simulink model "chapter2_5"  (R2022a) (continued)
== system chapter2_5/Subsystem3/Subsystem/Subsystem ==
  In1 : Inport
  In2 : Inport  Port=2
  Add : Sum  Inputs=++++++++++++++++++++
  Add Constant{,1..18} (19 alike) : Bias  Bias=1  SaturateOnIntegerOverflow=off
  Subsystem1 : Subsystem
  Subsystem10 : Subsystem
  Subsystem11 : Subsystem
  Subsystem12 : Subsystem
  Subsystem13 : Subsystem
  Subsystem14 : Subsystem
  Subsystem15 : Subsystem
  Subsystem16 : Subsystem
  Subsystem17 : Subsystem
  Subsystem18 : Subsystem
  Subsystem19 : Subsystem
  Subsystem2 : Subsystem
  Subsystem20 : Subsystem
  Subsystem3 : Subsystem
  Subsystem4 : Subsystem
  Subsystem5 : Subsystem
  Subsystem6 : Subsystem
  Subsystem7 : Subsystem
  Subsystem8 : Subsystem
  Subsystem9 : Subsystem
  Out1 : Outport
  wires:
  Subsystem14/1(Out1) -> Add/9
  Add Constant9/1 -> Subsystem11/1(index), Add Constant10/1
  Add Constant2/1 -> Subsystem2/1(index), Add Constant3/1
  [Ff] -> Subsystem13/2(F)
  Add Constant11/1 -> Subsystem16/1(index), Add Constant12/1
  Add Constant14/1 -> Subsystem13/1(index), Add Constant15/1
  Subsystem8/1(Out1) -> Add/5
  [Ff] -> Subsystem17/2(F)
  Add Constant15/1 -> Subsystem20/1(index), Add Constant16/1
  Subsystem1/1(Out1) -> Add/2
  Add Constant/1 -> Subsystem1/1(index), Add Constant1/1
  Add Constant3/1 -> Subsystem8/1(index), Add Constant4/1
  Subsystem9/1(Out1) -> Add/10
  [Ff] -> Subsystem3/2(F)
  Add Constant1/1 -> Subsystem3/1(index), Add Constant2/1
  Subsystem7/1(Out1) -> Add/7
  Add Constant17/1 -> Subsystem19/1(index), Add Constant18/1
  Add Constant18/1 -> Subsystem18/1(index)
  [Ff] -> Subsystem15/2(F)
  [Ff] -> Subsystem10/2(F)
  Subsystem5/1(Out1) -> Add/8
  Subsystem10/1(Out1) -> Add/12
  Subsystem6/1(Out1) -> Add/1
  Add Constant16/1 -> Subsystem17/1(index), Add Constant17/1
  Add Constant10/1 -> Subsystem10/1(index), Add Constant11/1
  [Ff] -> Subsystem20/2(F)
  Subsystem17/1(Out1) -> Add/18
  Subsystem19/1(Out1) -> Add/19
  [Ff] -> Subsystem1/2(F)
  [Ff] -> Subsystem19/2(F)
  Add Constant8/1 -> Subsystem9/1(index), Add Constant9/1
  [Ff] -> Subsystem2/2(F)
  Subsystem3/1(Out1) -> Add/3
  [Ff] -> Subsystem8/2(F)
  Subsystem20/1(Out1) -> Add/17
  Subsystem12/1(Out1) -> Add/14
  In1/1 -> Subsystem6/1(index), Add Constant/1
  Subsystem16/1(Out1) -> Add/13
  Subsystem13/1(Out1) -> Add/16
  [Ff] -> Subsystem6/2(F)
  [Ff] -> Subsystem7/2(F)
  [Ff] -> Subsystem11/2(F)
  Subsystem15/1(Out1) -> Add/15
  Add Constant13/1 -> Subsystem15/1(index), Add Constant14/1
  Subsystem2/1(Out1) -> Add/4
  Add Constant12/1 -> Subsystem12/1(index), Add Constant13/1
  Subsystem4/1(Out1) -> Add/6
  [Ff] -> Subsystem4/2(F)
  [Ff] -> Subsystem9/2(F)
  [Ff] -> Subsystem5/2(F)
  [Ff] -> Subsystem14/2(F)
  [Ff] -> Subsystem12/2(F)
  Subsystem18/1(Out1) -> Add/20
  Subsystem11/1(Out1) -> Add/11
  Add Constant5/1 -> Subsystem7/1(index), Add Constant6/1
  Add Constant6/1 -> Subsystem5/1(index), Add Constant7/1
  Add Constant4/1 -> Subsystem4/1(index), Add Constant5/1
  Add Constant7/1 -> Subsystem14/1(index), Add Constant8/1
  [Ff] -> Subsystem16/2(F)
  [Ff] -> Subsystem18/2(F)
  Add/1 -> Out1/1
  In2/1 -> [Ff]

== system chapter2_5/Subsystem3/Subsystem/Subsystem1 ==
  In1 : Inport
  In2 : Inport  Port=2
  Add : Sum  Inputs=++++++++++++++++++++
  Add Constant{,1..18} (19 alike) : Bias  Bias=1  SaturateOnIntegerOverflow=off
  Subsystem1 : Subsystem
  Subsystem10 : Subsystem
  Subsystem11 : Subsystem
  Subsystem12 : Subsystem
  Subsystem13 : Subsystem
  Subsystem14 : Subsystem
  Subsystem15 : Subsystem
  Subsystem16 : Subsystem
  Subsystem17 : Subsystem
  Subsystem18 : Subsystem
  Subsystem19 : Subsystem
  Subsystem2 : Subsystem
  Subsystem20 : Subsystem
  Subsystem3 : Subsystem
  Subsystem4 : Subsystem
  Subsystem5 : Subsystem
  Subsystem6 : Subsystem
  Subsystem7 : Subsystem
  Subsystem8 : Subsystem
  Subsystem9 : Subsystem
  Out1 : Outport
  wires:
  In2/1 -> [Ff]
  Add/1 -> Out1/1
  [Ff] -> Subsystem18/2(F)
  [Ff] -> Subsystem16/2(F)
  Add Constant7/1 -> Add Constant8/1, Subsystem14/1(index)
  Add Constant4/1 -> Add Constant5/1, Subsystem4/1(index)
  Add Constant6/1 -> Add Constant7/1, Subsystem5/1(index)
  Add Constant5/1 -> Add Constant6/1, Subsystem7/1(index)
  Subsystem11/1(Out1) -> Add/11
  Subsystem18/1(Out1) -> Add/20
  [Ff] -> Subsystem12/2(F)
  [Ff] -> Subsystem14/2(F)
  [Ff] -> Subsystem5/2(F)
  [Ff] -> Subsystem9/2(F)
  [Ff] -> Subsystem4/2(F)
  Subsystem4/1(Out1) -> Add/6
  Add Constant12/1 -> Add Constant13/1, Subsystem12/1(index)
  Subsystem2/1(Out1) -> Add/4
  Add Constant13/1 -> Add Constant14/1, Subsystem15/1(index)
  Subsystem15/1(Out1) -> Add/15
  [Ff] -> Subsystem11/2(F)
  [Ff] -> Subsystem7/2(F)
  [Ff] -> Subsystem6/2(F)
  Subsystem13/1(Out1) -> Add/16
  Subsystem16/1(Out1) -> Add/13
  In1/1 -> Add Constant/1, Subsystem6/1(index)
  Subsystem12/1(Out1) -> Add/14
  Subsystem20/1(Out1) -> Add/17
  [Ff] -> Subsystem8/2(F)
  Subsystem3/1(Out1) -> Add/3
  [Ff] -> Subsystem2/2(F)
  Add Constant8/1 -> Add Constant9/1, Subsystem9/1(index)
  [Ff] -> Subsystem19/2(F)
  [Ff] -> Subsystem1/2(F)
  Subsystem19/1(Out1) -> Add/19
  Subsystem17/1(Out1) -> Add/18
  [Ff] -> Subsystem20/2(F)
  Add Constant10/1 -> Add Constant11/1, Subsystem10/1(index)
  Add Constant16/1 -> Add Constant17/1, Subsystem17/1(index)
  Subsystem6/1(Out1) -> Add/1
  Subsystem10/1(Out1) -> Add/12
  Subsystem5/1(Out1) -> Add/8
  [Ff] -> Subsystem10/2(F)
  [Ff] -> Subsystem15/2(F)
  Add Constant18/1 -> Subsystem18/1(index)
  Add Constant17/1 -> Add Constant18/1, Subsystem19/1(index)
  Subsystem7/1(Out1) -> Add/7
  Add Constant1/1 -> Add Constant2/1, Subsystem3/1(index)
  [Ff] -> Subsystem3/2(F)
  Subsystem9/1(Out1) -> Add/10
  Add Constant3/1 -> Add Constant4/1, Subsystem8/1(index)
  Add Constant/1 -> Add Constant1/1, Subsystem1/1(index)
  Subsystem1/1(Out1) -> Add/2
  Add Constant15/1 -> Add Constant16/1, Subsystem20/1(index)
  [Ff] -> Subsystem17/2(F)
  Subsystem8/1(Out1) -> Add/5
  Add Constant14/1 -> Add Constant15/1, Subsystem13/1(index)
  Add Constant11/1 -> Add Constant12/1, Subsystem16/1(index)
  [Ff] -> Subsystem13/2(F)
  Add Constant2/1 -> Add Constant3/1, Subsystem2/1(index)
  Add Constant9/1 -> Add Constant10/1, Subsystem11/1(index)
  Subsystem14/1(Out1) -> Add/9

== system chapter2_5/Subsystem3/Subsystem/Subsystem2 ==
  In1 : Inport
  In2 : Inport  Port=2
  Add : Sum  Inputs=++++++++++++++++++++
  Add Constant{,1..18} (19 alike) : Bias  Bias=1  SaturateOnIntegerOverflow=off
  Subsystem1 : Subsystem
  Subsystem10 : Subsystem
  Subsystem11 : Subsystem
  Subsystem12 : Subsystem
  Subsystem13 : Subsystem
  Subsystem14 : Subsystem
  Subsystem15 : Subsystem
  Subsystem16 : Subsystem
  Subsystem17 : Subsystem
  Subsystem18 : Subsystem
  Subsystem19 : Subsystem
  Subsystem2 : Subsystem
  Subsystem20 : Subsystem
  Subsystem3 : Subsystem
  Subsystem4 : Subsystem
  Subsystem5 : Subsystem
  Subsystem6 : Subsystem
  Subsystem7 : Subsystem
  Subsystem8 : Subsystem
  Subsystem9 : Subsystem
  Out1 : Outport
  wires:
  Subsystem14/1(Out1) -> Add/9
  Add Constant9/1 -> Subsystem11/1(index), Add Constant10/1
  Add Constant2/1 -> Subsystem2/1(index), Add Constant3/1
  [Ff] -> Subsystem13/2(F)
  Add Constant11/1 -> Subsystem16/1(index), Add Constant12/1
  Add Constant14/1 -> Subsystem13/1(index), Add Constant15/1
  Subsystem8/1(Out1) -> Add/5
  [Ff] -> Subsystem17/2(F)
  Add Constant15/1 -> Subsystem20/1(index), Add Constant16/1
  Subsystem1/1(Out1) -> Add/2
  Add Constant/1 -> Subsystem1/1(index), Add Constant1/1
  Add Constant3/1 -> Subsystem8/1(index), Add Constant4/1
  Subsystem9/1(Out1) -> Add/10
  [Ff] -> Subsystem3/2(F)
  Add Constant1/1 -> Subsystem3/1(index), Add Constant2/1
  Subsystem7/1(Out1) -> Add/7
  Add Constant17/1 -> Subsystem19/1(index), Add Constant18/1
  Add Constant18/1 -> Subsystem18/1(index)
  [Ff] -> Subsystem15/2(F)
  [Ff] -> Subsystem10/2(F)
  Subsystem5/1(Out1) -> Add/8
  Subsystem10/1(Out1) -> Add/12
  Subsystem6/1(Out1) -> Add/1
  Add Constant16/1 -> Subsystem17/1(index), Add Constant17/1
  Add Constant10/1 -> Subsystem10/1(index), Add Constant11/1
  [Ff] -> Subsystem20/2(F)
  Subsystem17/1(Out1) -> Add/18
  Subsystem19/1(Out1) -> Add/19
  [Ff] -> Subsystem1/2(F)
  [Ff] -> Subsystem19/2(F)
  Add Constant8/1 -> Subsystem9/1(index), Add Constant9/1
  [Ff] -> Subsystem2/2(F)
  Subsystem3/1(Out1) -> Add/3
  [Ff] -> Subsystem8/2(F)
  Subsystem20/1(Out1) -> Add/17
  Subsystem12/1(Out1) -> Add/14
  In1/1 -> Subsystem6/1(index), Add Constant/1
  Subsystem16/1(Out1) -> Add/13
  Subsystem13/1(Out1) -> Add/16
  [Ff] -> Subsystem6/2(F)
  [Ff] -> Subsystem7/2(F)
  [Ff] -> Subsystem11/2(F)
  Subsystem15/1(Out1) -> Add/15
  Add Constant13/1 -> Subsystem15/1(index), Add Constant14/1
  Subsystem2/1(Out1) -> Add/4
  Add Constant12/1 -> Subsystem12/1(index), Add Constant13/1
  Subsystem4/1(Out1) -> Add/6
  [Ff] -> Subsystem4/2(F)
  [Ff] -> Subsystem9/2(F)
  [Ff] -> Subsystem5/2(F)
  [Ff] -> Subsystem14/2(F)
  [Ff] -> Subsystem12/2(F)
  Subsystem18/1(Out1) -> Add/20
  Subsystem11/1(Out1) -> Add/11
  Add Constant5/1 -> Subsystem7/1(index), Add Constant6/1
  Add Constant6/1 -> Subsystem5/1(index), Add Constant7/1
  Add Constant4/1 -> Subsystem4/1(index), Add Constant5/1
  Add Constant7/1 -> Subsystem14/1(index), Add Constant8/1
  [Ff] -> Subsystem16/2(F)
  [Ff] -> Subsystem18/2(F)
  Add/1 -> Out1/1
  In2/1 -> [Ff]

== system chapter2_5/Subsystem3/Subsystem/Subsystem3 ==
  In1 : Inport
  In2 : Inport  Port=2
  Add : Sum  Inputs=++++++++++++++++++++
  Add Constant{,1..18} (19 alike) : Bias  Bias=1  SaturateOnIntegerOverflow=off
  Subsystem1 : Subsystem
  Subsystem10 : Subsystem
  Subsystem11 : Subsystem
  Subsystem12 : Subsystem
  Subsystem13 : Subsystem
  Subsystem14 : Subsystem
  Subsystem15 : Subsystem
  Subsystem16 : Subsystem
  Subsystem17 : Subsystem
  Subsystem18 : Subsystem
  Subsystem19 : Subsystem
  Subsystem2 : Subsystem
  Subsystem20 : Subsystem
  Subsystem3 : Subsystem
  Subsystem4 : Subsystem
  Subsystem5 : Subsystem
  Subsystem6 : Subsystem
  Subsystem7 : Subsystem
  Subsystem8 : Subsystem
  Subsystem9 : Subsystem
  Out1 : Outport
  wires:
  In2/1 -> [Ff]
  Add/1 -> Out1/1
  [Ff] -> Subsystem18/2(F)
  [Ff] -> Subsystem16/2(F)
  Add Constant7/1 -> Add Constant8/1, Subsystem14/1(index)
  Add Constant4/1 -> Add Constant5/1, Subsystem4/1(index)
  Add Constant6/1 -> Add Constant7/1, Subsystem5/1(index)
  Add Constant5/1 -> Add Constant6/1, Subsystem7/1(index)
  Subsystem11/1(Out1) -> Add/11
  Subsystem18/1(Out1) -> Add/20
  [Ff] -> Subsystem12/2(F)
  [Ff] -> Subsystem14/2(F)
  [Ff] -> Subsystem5/2(F)
  [Ff] -> Subsystem9/2(F)
  [Ff] -> Subsystem4/2(F)
  Subsystem4/1(Out1) -> Add/6
  Add Constant12/1 -> Add Constant13/1, Subsystem12/1(index)
  Subsystem2/1(Out1) -> Add/4
  Add Constant13/1 -> Add Constant14/1, Subsystem15/1(index)
  Subsystem15/1(Out1) -> Add/15
  [Ff] -> Subsystem11/2(F)
  [Ff] -> Subsystem7/2(F)
  [Ff] -> Subsystem6/2(F)
  Subsystem13/1(Out1) -> Add/16
  Subsystem16/1(Out1) -> Add/13
  In1/1 -> Add Constant/1, Subsystem6/1(index)
  Subsystem12/1(Out1) -> Add/14
  Subsystem20/1(Out1) -> Add/17
  [Ff] -> Subsystem8/2(F)
  Subsystem3/1(Out1) -> Add/3
  [Ff] -> Subsystem2/2(F)
  Add Constant8/1 -> Add Constant9/1, Subsystem9/1(index)
  [Ff] -> Subsystem19/2(F)
  [Ff] -> Subsystem1/2(F)
  Subsystem19/1(Out1) -> Add/19
  Subsystem17/1(Out1) -> Add/18
  [Ff] -> Subsystem20/2(F)
  Add Constant10/1 -> Add Constant11/1, Subsystem10/1(index)
  Add Constant16/1 -> Add Constant17/1, Subsystem17/1(index)
  Subsystem6/1(Out1) -> Add/1
  Subsystem10/1(Out1) -> Add/12
  Subsystem5/1(Out1) -> Add/8
  [Ff] -> Subsystem10/2(F)
  [Ff] -> Subsystem15/2(F)
  Add Constant18/1 -> Subsystem18/1(index)
  Add Constant17/1 -> Add Constant18/1, Subsystem19/1(index)
  Subsystem7/1(Out1) -> Add/7
  Add Constant1/1 -> Add Constant2/1, Subsystem3/1(index)
  [Ff] -> Subsystem3/2(F)
  Subsystem9/1(Out1) -> Add/10
  Add Constant3/1 -> Add Constant4/1, Subsystem8/1(index)
  Add Constant/1 -> Add Constant1/1, Subsystem1/1(index)
  Subsystem1/1(Out1) -> Add/2
  Add Constant15/1 -> Add Constant16/1, Subsystem20/1(index)
  [Ff] -> Subsystem17/2(F)
  Subsystem8/1(Out1) -> Add/5
  Add Constant14/1 -> Add Constant15/1, Subsystem13/1(index)
  Add Constant11/1 -> Add Constant12/1, Subsystem16/1(index)
  [Ff] -> Subsystem13/2(F)
  Add Constant2/1 -> Add Constant3/1, Subsystem2/1(index)
  Add Constant9/1 -> Add Constant10/1, Subsystem11/1(index)
  Subsystem14/1(Out1) -> Add/9

== system chapter2_5/Subsystem3/Subsystem/Subsystem4 ==
  In1 : Inport
  In2 : Inport  Port=2
  Add : Sum  Inputs=++++++++++++++++++++
  Add Constant{,1..18} (19 alike) : Bias  Bias=1  SaturateOnIntegerOverflow=off
  Subsystem1 : Subsystem
  Subsystem10 : Subsystem
  Subsystem11 : Subsystem
  Subsystem12 : Subsystem
  Subsystem13 : Subsystem
  Subsystem14 : Subsystem
  Subsystem15 : Subsystem
  Subsystem16 : Subsystem
  Subsystem17 : Subsystem
  Subsystem18 : Subsystem
  Subsystem19 : Subsystem
  Subsystem2 : Subsystem
  Subsystem20 : Subsystem
  Subsystem3 : Subsystem
  Subsystem4 : Subsystem
  Subsystem5 : Subsystem
  Subsystem6 : Subsystem
  Subsystem7 : Subsystem
  Subsystem8 : Subsystem
  Subsystem9 : Subsystem
  Out1 : Outport
  wires:
  Subsystem14/1(Out1) -> Add/9
  Add Constant9/1 -> Subsystem11/1(index), Add Constant10/1
  Add Constant2/1 -> Subsystem2/1(index), Add Constant3/1
  [Ff] -> Subsystem13/2(F)
  Add Constant11/1 -> Subsystem16/1(index), Add Constant12/1
  Add Constant14/1 -> Subsystem13/1(index), Add Constant15/1
  Subsystem8/1(Out1) -> Add/5
  [Ff] -> Subsystem17/2(F)
  Add Constant15/1 -> Subsystem20/1(index), Add Constant16/1
  Subsystem1/1(Out1) -> Add/2
  Add Constant/1 -> Subsystem1/1(index), Add Constant1/1
  Add Constant3/1 -> Subsystem8/1(index), Add Constant4/1
  Subsystem9/1(Out1) -> Add/10
  [Ff] -> Subsystem3/2(F)
  Add Constant1/1 -> Subsystem3/1(index), Add Constant2/1
  Subsystem7/1(Out1) -> Add/7
  Add Constant17/1 -> Subsystem19/1(index), Add Constant18/1
  Add Constant18/1 -> Subsystem18/1(index)
  [Ff] -> Subsystem15/2(F)
  [Ff] -> Subsystem10/2(F)
  Subsystem5/1(Out1) -> Add/8
  Subsystem10/1(Out1) -> Add/12
  Subsystem6/1(Out1) -> Add/1
  Add Constant16/1 -> Subsystem17/1(index), Add Constant17/1
  Add Constant10/1 -> Subsystem10/1(index), Add Constant11/1
  [Ff] -> Subsystem20/2(F)
  Subsystem17/1(Out1) -> Add/18
  Subsystem19/1(Out1) -> Add/19
  [Ff] -> Subsystem1/2(F)
  [Ff] -> Subsystem19/2(F)
  Add Constant8/1 -> Subsystem9/1(index), Add Constant9/1
  [Ff] -> Subsystem2/2(F)
  Subsystem3/1(Out1) -> Add/3
  [Ff] -> Subsystem8/2(F)
  Subsystem20/1(Out1) -> Add/17
  Subsystem12/1(Out1) -> Add/14
  In1/1 -> Subsystem6/1(index), Add Constant/1
  Subsystem16/1(Out1) -> Add/13
  Subsystem13/1(Out1) -> Add/16
  [Ff] -> Subsystem6/2(F)
  [Ff] -> Subsystem7/2(F)
  [Ff] -> Subsystem11/2(F)
  Subsystem15/1(Out1) -> Add/15
  Add Constant13/1 -> Subsystem15/1(index), Add Constant14/1
  Subsystem2/1(Out1) -> Add/4
  Add Constant12/1 -> Subsystem12/1(index), Add Constant13/1
  Subsystem4/1(Out1) -> Add/6
  [Ff] -> Subsystem4/2(F)
  [Ff] -> Subsystem9/2(F)
  [Ff] -> Subsystem5/2(F)
  [Ff] -> Subsystem14/2(F)
  [Ff] -> Subsystem12/2(F)
  Subsystem18/1(Out1) -> Add/20
  Subsystem11/1(Out1) -> Add/11
  Add Constant5/1 -> Subsystem7/1(index), Add Constant6/1
  Add Constant6/1 -> Subsystem5/1(index), Add Constant7/1
  Add Constant4/1 -> Subsystem4/1(index), Add Constant5/1
  Add Constant7/1 -> Subsystem14/1(index), Add Constant8/1
  [Ff] -> Subsystem16/2(F)
  [Ff] -> Subsystem18/2(F)
  Add/1 -> Out1/1
  In2/1 -> [Ff]

== system chapter2_5/Subsystem3/Subsystem/Subsystem5 ==
  In1 : Inport
  In2 : Inport  Port=2
  Add : Sum  Inputs=++++++++++++++++++++
  Add Constant{,1..18} (19 alike) : Bias  Bias=1  SaturateOnIntegerOverflow=off
  Subsystem1 : Subsystem
  Subsystem10 : Subsystem
  Subsystem11 : Subsystem
  Subsystem12 : Subsystem
  Subsystem13 : Subsystem
  Subsystem14 : Subsystem
  Subsystem15 : Subsystem
  Subsystem16 : Subsystem
  Subsystem17 : Subsystem
  Subsystem18 : Subsystem
  Subsystem19 : Subsystem
  Subsystem2 : Subsystem
  Subsystem20 : Subsystem
  Subsystem3 : Subsystem
  Subsystem4 : Subsystem
  Subsystem5 : Subsystem
  Subsystem6 : Subsystem
  Subsystem7 : Subsystem
  Subsystem8 : Subsystem
  Subsystem9 : Subsystem
  Out1 : Outport
  wires:
  In2/1 -> [Ff]
  Add/1 -> Out1/1
  [Ff] -> Subsystem18/2(F)
  [Ff] -> Subsystem16/2(F)
  Add Constant7/1 -> Add Constant8/1, Subsystem14/1(index)
  Add Constant4/1 -> Add Constant5/1, Subsystem4/1(index)
  Add Constant6/1 -> Add Constant7/1, Subsystem5/1(index)
  Add Constant5/1 -> Add Constant6/1, Subsystem7/1(index)
  Subsystem11/1(Out1) -> Add/11
  Subsystem18/1(Out1) -> Add/20
  [Ff] -> Subsystem12/2(F)
  [Ff] -> Subsystem14/2(F)
  [Ff] -> Subsystem5/2(F)
  [Ff] -> Subsystem9/2(F)
  [Ff] -> Subsystem4/2(F)
  Subsystem4/1(Out1) -> Add/6
  Add Constant12/1 -> Add Constant13/1, Subsystem12/1(index)
  Subsystem2/1(Out1) -> Add/4
  Add Constant13/1 -> Add Constant14/1, Subsystem15/1(index)
  Subsystem15/1(Out1) -> Add/15
  [Ff] -> Subsystem11/2(F)
  [Ff] -> Subsystem7/2(F)
  [Ff] -> Subsystem6/2(F)
  Subsystem13/1(Out1) -> Add/16
  Subsystem16/1(Out1) -> Add/13
  In1/1 -> Add Constant/1, Subsystem6/1(index)
  Subsystem12/1(Out1) -> Add/14
  Subsystem20/1(Out1) -> Add/17
  [Ff] -> Subsystem8/2(F)
  Subsystem3/1(Out1) -> Add/3
  [Ff] -> Subsystem2/2(F)
  Add Constant8/1 -> Add Constant9/1, Subsystem9/1(index)
  [Ff] -> Subsystem19/2(F)
  [Ff] -> Subsystem1/2(F)
  Subsystem19/1(Out1) -> Add/19
  Subsystem17/1(Out1) -> Add/18
  [Ff] -> Subsystem20/2(F)
  Add Constant10/1 -> Add Constant11/1, Subsystem10/1(index)
  Add Constant16/1 -> Add Constant17/1, Subsystem17/1(index)
  Subsystem6/1(Out1) -> Add/1
  Subsystem10/1(Out1) -> Add/12
  Subsystem5/1(Out1) -> Add/8
  [Ff] -> Subsystem10/2(F)
  [Ff] -> Subsystem15/2(F)
  Add Constant18/1 -> Subsystem18/1(index)
  Add Constant17/1 -> Add Constant18/1, Subsystem19/1(index)
  Subsystem7/1(Out1) -> Add/7
  Add Constant1/1 -> Add Constant2/1, Subsystem3/1(index)
  [Ff] -> Subsystem3/2(F)
  Subsystem9/1(Out1) -> Add/10
  Add Constant3/1 -> Add Constant4/1, Subsystem8/1(index)
  Add Constant/1 -> Add Constant1/1, Subsystem1/1(index)
  Subsystem1/1(Out1) -> Add/2
  Add Constant15/1 -> Add Constant16/1, Subsystem20/1(index)
  [Ff] -> Subsystem17/2(F)
  Subsystem8/1(Out1) -> Add/5
  Add Constant14/1 -> Add Constant15/1, Subsystem13/1(index)
  Add Constant11/1 -> Add Constant12/1, Subsystem16/1(index)
  [Ff] -> Subsystem13/2(F)
  Add Constant2/1 -> Add Constant3/1, Subsystem2/1(index)
  Add Constant9/1 -> Add Constant10/1, Subsystem11/1(index)
  Subsystem14/1(Out1) -> Add/9

== system chapter2_5/Subsystem3/Subsystem/Subsystem6 ==
  In1 : Inport
  In2 : Inport  Port=2
  Add : Sum  Inputs=++++++++++++++++++++
  Add Constant{,1..18} (19 alike) : Bias  Bias=1  SaturateOnIntegerOverflow=off
  Subsystem1 : Subsystem
  Subsystem10 : Subsystem
  Subsystem11 : Subsystem
  Subsystem12 : Subsystem
  Subsystem13 : Subsystem
  Subsystem14 : Subsystem
  Subsystem15 : Subsystem
  Subsystem16 : Subsystem
  Subsystem17 : Subsystem
  Subsystem18 : Subsystem
  Subsystem19 : Subsystem
  Subsystem2 : Subsystem
  Subsystem20 : Subsystem
  Subsystem3 : Subsystem
  Subsystem4 : Subsystem
  Subsystem5 : Subsystem
  Subsystem6 : Subsystem
  Subsystem7 : Subsystem
  Subsystem8 : Subsystem
  Subsystem9 : Subsystem
  Out1 : Outport
  wires:
  Subsystem14/1(Out1) -> Add/9
  Add Constant9/1 -> Subsystem11/1(index), Add Constant10/1
  Add Constant2/1 -> Subsystem2/1(index), Add Constant3/1
  [Ff] -> Subsystem13/2(F)
  Add Constant11/1 -> Subsystem16/1(index), Add Constant12/1
  Add Constant14/1 -> Subsystem13/1(index), Add Constant15/1
  Subsystem8/1(Out1) -> Add/5
  [Ff] -> Subsystem17/2(F)
  Add Constant15/1 -> Subsystem20/1(index), Add Constant16/1
  Subsystem1/1(Out1) -> Add/2
  Add Constant/1 -> Subsystem1/1(index), Add Constant1/1
  Add Constant3/1 -> Subsystem8/1(index), Add Constant4/1
  Subsystem9/1(Out1) -> Add/10
  [Ff] -> Subsystem3/2(F)
  Add Constant1/1 -> Subsystem3/1(index), Add Constant2/1
  Subsystem7/1(Out1) -> Add/7
  Add Constant17/1 -> Subsystem19/1(index), Add Constant18/1
  Add Constant18/1 -> Subsystem18/1(index)
  [Ff] -> Subsystem15/2(F)
  [Ff] -> Subsystem10/2(F)
  Subsystem5/1(Out1) -> Add/8
  Subsystem10/1(Out1) -> Add/12
  Subsystem6/1(Out1) -> Add/1
  Add Constant16/1 -> Subsystem17/1(index), Add Constant17/1
  Add Constant10/1 -> Subsystem10/1(index), Add Constant11/1
  [Ff] -> Subsystem20/2(F)
  Subsystem17/1(Out1) -> Add/18
  Subsystem19/1(Out1) -> Add/19
  [Ff] -> Subsystem1/2(F)
  [Ff] -> Subsystem19/2(F)
  Add Constant8/1 -> Subsystem9/1(index), Add Constant9/1
  [Ff] -> Subsystem2/2(F)
  Subsystem3/1(Out1) -> Add/3
  [Ff] -> Subsystem8/2(F)
  Subsystem20/1(Out1) -> Add/17
  Subsystem12/1(Out1) -> Add/14
  In1/1 -> Subsystem6/1(index), Add Constant/1
  Subsystem16/1(Out1) -> Add/13
  Subsystem13/1(Out1) -> Add/16
  [Ff] -> Subsystem6/2(F)
  [Ff] -> Subsystem7/2(F)
  [Ff] -> Subsystem11/2(F)
  Subsystem15/1(Out1) -> Add/15
  Add Constant13/1 -> Subsystem15/1(index), Add Constant14/1
  Subsystem2/1(Out1) -> Add/4
  Add Constant12/1 -> Subsystem12/1(index), Add Constant13/1
  Subsystem4/1(Out1) -> Add/6
  [Ff] -> Subsystem4/2(F)
  [Ff] -> Subsystem9/2(F)
  [Ff] -> Subsystem5/2(F)
  [Ff] -> Subsystem14/2(F)
  [Ff] -> Subsystem12/2(F)
  Subsystem18/1(Out1) -> Add/20
  Subsystem11/1(Out1) -> Add/11
  Add Constant5/1 -> Subsystem7/1(index), Add Constant6/1
  Add Constant6/1 -> Subsystem5/1(index), Add Constant7/1
  Add Constant4/1 -> Subsystem4/1(index), Add Constant5/1
  Add Constant7/1 -> Subsystem14/1(index), Add Constant8/1
  [Ff] -> Subsystem16/2(F)
  [Ff] -> Subsystem18/2(F)
  Add/1 -> Out1/1
  In2/1 -> [Ff]

== system chapter2_5/Subsystem3/Subsystem1/Subsystem ==
  In1 : Inport
  In2 : Inport  Port=2
  Add : Sum  Inputs=++++++++++++++++++++
  Add Constant{,1..18} (19 alike) : Bias  Bias=1  SaturateOnIntegerOverflow=off
  Subsystem1 : Subsystem
  Subsystem10 : Subsystem
  Subsystem11 : Subsystem
  Subsystem12 : Subsystem
  Subsystem13 : Subsystem
  Subsystem14 : Subsystem
  Subsystem15 : Subsystem
  Subsystem16 : Subsystem
  Subsystem17 : Subsystem
  Subsystem18 : Subsystem
  Subsystem19 : Subsystem
  Subsystem2 : Subsystem
  Subsystem20 : Subsystem
  Subsystem3 : Subsystem
  Subsystem4 : Subsystem
  Subsystem5 : Subsystem
  Subsystem6 : Subsystem
  Subsystem7 : Subsystem
  Subsystem8 : Subsystem
  Subsystem9 : Subsystem
  Out1 : Outport
  wires:
  Add Constant7/1 -> Subsystem13/1(index), Add Constant8/1
  Subsystem15/1(Out1) -> Add/15
  [Ff] -> Subsystem3/2(F)
  Add Constant14/1 -> Subsystem12/1(index), Add Constant15/1
  Subsystem8/1(Out1) -> Add/5
  [Ff] -> Subsystem7/2(F)
  Add Constant8/1 -> Subsystem9/1(index), Add Constant9/1
  Subsystem9/1(Out1) -> Add/10
  [Ff] -> Subsystem6/2(F)
  Add Constant4/1 -> Subsystem4/1(index), Add Constant5/1
  Subsystem18/1(Out1) -> Add/20
  Add Constant15/1 -> Subsystem15/1(index), Add Constant16/1
  Subsystem4/1(Out1) -> Add/6
  Add Constant6/1 -> Subsystem6/1(index), Add Constant7/1
  Subsystem16/1(Out1) -> Add/13
  In1/1 -> Add Constant/1, Subsystem5/1(index)
  Subsystem20/1(Out1) -> Add/17
  [Ff] -> Subsystem17/2(F)
  [Ff] -> Subsystem10/2(F)
  Add Constant10/1 -> Subsystem10/1(index), Add Constant11/1
  Add Constant12/1 -> Subsystem17/1(index), Add Constant13/1
  Add Constant3/1 -> Subsystem8/1(index), Add Constant4/1
  Add Constant/1 -> Subsystem1/1(index), Add Constant1/1
  [Ff] -> Subsystem11/2(F)
  Subsystem11/1(Out1) -> Add/11
  [Ff] -> Subsystem19/2(F)
  Subsystem5/1(Out1) -> Add/1
  [Ff] -> Subsystem15/2(F)
  Subsystem1/1(Out1) -> Add/2
  Add Constant13/1 -> Subsystem19/1(index), Add Constant18/1
  [Ff] -> Subsystem9/2(F)
  Add Constant1/1 -> Subsystem3/1(index), Add Constant2/1
  Subsystem10/1(Out1) -> Add/12
  Add Constant2/1 -> Subsystem2/1(index), Add Constant3/1
  [Ff] -> Subsystem2/2(F)
  Subsystem12/1(Out1) -> Add/14
  [Ff] -> Subsystem18/2(F)
  [Ff] -> Subsystem4/2(F)
  Subsystem17/1(Out1) -> Add/18
  Subsystem13/1(Out1) -> Add/9
  [Ff] -> Subsystem1/2(F)
  [Ff] -> Subsystem16/2(F)
  Subsystem14/1(Out1) -> Add/16
  [Ff] -> Subsystem5/2(F)
  [Ff] -> Subsystem20/2(F)
  Subsystem3/1(Out1) -> Add/3
  Subsystem7/1(Out1) -> Add/7
  Add/1 -> Out1/1
  [Ff] -> Subsystem14/2(F)
  [Ff] -> Subsystem8/2(F)
  Subsystem19/1(Out1) -> Add/19
  [Ff] -> Subsystem12/2(F)
  [Ff] -> Subsystem13/2(F)
  Add Constant9/1 -> Subsystem11/1(index), Add Constant10/1
  Add Constant5/1 -> Subsystem7/1(index), Add Constant6/1
  Subsystem6/1(Out1) -> Add/8
  Add Constant11/1 -> Subsystem16/1(index), Add Constant14/1
  Subsystem2/1(Out1) -> Add/4
  Add Constant16/1 -> Subsystem14/1(index), Add Constant17/1
  Add Constant17/1 -> Subsystem20/1(index), Add Constant12/1
  Add Constant18/1 -> Subsystem18/1(index)
  In2/1 -> [Ff]

== system chapter2_5/Subsystem3/Subsystem1/Subsystem1 ==
  In1 : Inport
  In2 : Inport  Port=2
  Add : Sum  Inputs=++++++++++++++++++++
  Add Constant{,1..18} (19 alike) : Bias  Bias=1  SaturateOnIntegerOverflow=off
  Subsystem1 : Subsystem
  Subsystem10 : Subsystem
  Subsystem11 : Subsystem
  Subsystem12 : Subsystem
  Subsystem13 : Subsystem
  Subsystem14 : Subsystem
  Subsystem15 : Subsystem
  Subsystem16 : Subsystem
  Subsystem17 : Subsystem
  Subsystem18 : Subsystem
  Subsystem19 : Subsystem
  Subsystem2 : Subsystem
  Subsystem20 : Subsystem
  Subsystem3 : Subsystem
  Subsystem4 : Subsystem
  Subsystem5 : Subsystem
  Subsystem6 : Subsystem
  Subsystem7 : Subsystem
  Subsystem8 : Subsystem
  Subsystem9 : Subsystem
  Out1 : Outport
  wires:
  In2/1 -> [Ff]
  Add Constant18/1 -> Subsystem18/1(index)
  Add Constant17/1 -> Add Constant12/1, Subsystem20/1(index)
  Add Constant16/1 -> Add Constant17/1, Subsystem14/1(index)
  Subsystem2/1(Out1) -> Add/4
  Add Constant11/1 -> Add Constant14/1, Subsystem16/1(index)
  Subsystem6/1(Out1) -> Add/8
  Add Constant5/1 -> Add Constant6/1, Subsystem7/1(index)
  Add Constant9/1 -> Add Constant10/1, Subsystem11/1(index)
  [Ff] -> Subsystem13/2(F)
  [Ff] -> Subsystem12/2(F)
  Subsystem19/1(Out1) -> Add/19
  [Ff] -> Subsystem8/2(F)
  [Ff] -> Subsystem14/2(F)
  Add/1 -> Out1/1
  Subsystem7/1(Out1) -> Add/7
  Subsystem3/1(Out1) -> Add/3
  [Ff] -> Subsystem20/2(F)
  [Ff] -> Subsystem5/2(F)
  Subsystem14/1(Out1) -> Add/16
  [Ff] -> Subsystem16/2(F)
  [Ff] -> Subsystem1/2(F)
  Subsystem13/1(Out1) -> Add/9
  Subsystem17/1(Out1) -> Add/18
  [Ff] -> Subsystem4/2(F)
  [Ff] -> Subsystem18/2(F)
  Subsystem12/1(Out1) -> Add/14
  [Ff] -> Subsystem2/2(F)
  Add Constant2/1 -> Add Constant3/1, Subsystem2/1(index)
  Subsystem10/1(Out1) -> Add/12
  Add Constant1/1 -> Add Constant2/1, Subsystem3/1(index)
  [Ff] -> Subsystem9/2(F)
  Add Constant13/1 -> Add Constant18/1, Subsystem19/1(index)
  Subsystem1/1(Out1) -> Add/2
  [Ff] -> Subsystem15/2(F)
  Subsystem5/1(Out1) -> Add/1
  [Ff] -> Subsystem19/2(F)
  Subsystem11/1(Out1) -> Add/11
  [Ff] -> Subsystem11/2(F)
  Add Constant/1 -> Add Constant1/1, Subsystem1/1(index)
  Add Constant3/1 -> Add Constant4/1, Subsystem8/1(index)
  Add Constant12/1 -> Add Constant13/1, Subsystem17/1(index)
  Add Constant10/1 -> Add Constant11/1, Subsystem10/1(index)
  [Ff] -> Subsystem10/2(F)
  [Ff] -> Subsystem17/2(F)
  Subsystem20/1(Out1) -> Add/17
  In1/1 -> Subsystem5/1(index), Add Constant/1
  Subsystem16/1(Out1) -> Add/13
  Add Constant6/1 -> Add Constant7/1, Subsystem6/1(index)
  Subsystem4/1(Out1) -> Add/6
  Add Constant15/1 -> Add Constant16/1, Subsystem15/1(index)
  Subsystem18/1(Out1) -> Add/20
  Add Constant4/1 -> Add Constant5/1, Subsystem4/1(index)
  [Ff] -> Subsystem6/2(F)
  Subsystem9/1(Out1) -> Add/10
  Add Constant8/1 -> Add Constant9/1, Subsystem9/1(index)
  [Ff] -> Subsystem7/2(F)
  Subsystem8/1(Out1) -> Add/5
  Add Constant14/1 -> Add Constant15/1, Subsystem12/1(index)
  [Ff] -> Subsystem3/2(F)
  Subsystem15/1(Out1) -> Add/15
  Add Constant7/1 -> Add Constant8/1, Subsystem13/1(index)
